simulink model "TosAeb"  (R2021a)
settings: Solver=FixedStepDiscrete  StartTime=0.0  StopTime=inf  FixedStep=0.01  RelTol=1e-3
undefined symbols (not defined in the file or in its folder): ACCModSts, AccSts1, AEB_ObjectMovingOrientation, AEB_ObjectMovingState, CN_BTCActive, CN_CurrentGearR, CN_CurrentGearR_DHT, CN_PTCActive, CN_VDCActive, CN_VSC_EspOff, CN_VSC_FCW_AEB_Error, CN_VSC_FCW_AEB_NoDispay, CN_VSC_FCW_AEB_PerfmDgdtn, CN_VSC_FCW_AEB_TempUnAvlbl, CN_VSC_VehStandstill, CNaccelAvoidMax, CNaccelAvoidMin, CNbrakePedalPress, CNcomfortStrLeftAccelMax, CNcomfortStrLeftAccelMin, CNcomfortStrRightAccelMax, CNcomfortStrRightAccelMin, CNDrvDoorOpen, CNDrvModeEco, CNDrvModeNorm, CNDrvModeOther, CNDrvModeSport, CNDrvSBRreminder, CNNull, CNobjInvalidID, CNPassDoorOpen, CNPassSBRreminder, CNPEBSAccelNegInf, CNPEBSAccelNull, CNPEBSTimeInf, CNPEBSTimeNull, CNSTOPMAX, CNSTOPNULL, CNsubjWidth, CNSwtOFF, CNSwtON, CNTTCMAX, CNTTCNULL, CNVehConfigAEBCycNone, CNVehConfigAEBNone, CNVehConfigAEBPedNone, CNVehConfigFCWNone, CNVehConfigJANone, CollisionSeverity, FALSE, FCWSnvty, JACycObjCalcInfoDefaultParams, JAPedObjCalcInfoDefaultParams, JAVehObjCalcInfoDefaultParams, latCycObjCalcInfoDefaultParams, latPedObjCalcInfoDefaultParams, longObjCalcInfoDefaultParams, ModSts, movingStatus, ObjectType ... (+1112)
libraries: simulink x3031, AEBSFunctionLib x50, Ta_Library x33, AEBSStateMachineLib x23, Mylibrary x12, simulink_extras x7, ObjSelect_lib x3, dspstat3 x2
(part 55 of 102)

== system TosAeb/FcwAebJa_Step_sys/ALG_AEBSystem/ALG_AEBController/AEB_FuncImpManagementApp/FuncManeuArbCalc/brkFuncManeuArbCalc/axTgtReqValueCalc1/axTgtReqValueCalc4AebControl/aDesiredCalc ==
  AebActiveFirstCycle : Inport
  subjVel : Inport  Port=2
  axAbsObj : Inport  Port=3
  dxFrontBumperPredicted : Inport  Port=4
  vxAbsObjPredicted : Inport  Port=5
  vxPredicted : Inport  Port=6
  dxSecureAtStart : Inport  Port=7
  accelMin : Inport  Port=8
  Bus Creator : BusCreator  Inputs=8
  Bus Selector : BusSelector  OutputSignals=dxFrontBumperPredicted,dxSecure,vxPredicted,subjVel
  Bus Selector1 : BusSelector  OutputSignals=vxPredicted,axAbsObj,vxAbsObjPredicted,dxFrontBumperPredicted,subjVel,dxSecure
  Bus Selector2 : BusSelector  OutputSignals=accelMin
  Bus Selector3 : BusSelector  OutputSignals=AebActiveFirstCycle
  If : If  IfExpression=u1
  If Action Subsystem : Subsystem  TreatAsAtomicUnit=on
  If Action Subsystem1 : Subsystem  TreatAsAtomicUnit=on
  Merge : Merge
  accelAvoidFilt : Subsystem
  underDxSecure : Subsystem
  aDesired : Outport
  wires:
  accelAvoidFilt/1(aDesired) -> aDesired/1
  Merge/1 -> accelAvoidFilt/2(accelAvoid)  "accelAvoidRaw"
  vxPredicted/1 -> Bus Creator/4  "vxPredicted"
  vxAbsObjPredicted/1 -> Bus Creator/3  "vxAbsObjPredicted"
  accelMin/1 -> Bus Creator/6  "accelMin"
  subjVel/1 -> Bus Creator/8  "subjVel"
  axAbsObj/1 -> Bus Creator/7  "axAbsObj"
  Bus Selector2/1 -> If Action Subsystem/1(accelMin)  "<accelMin>"
  Bus Selector/2 -> underDxSecure/2(dxSecure)  "<dxSecure>"
  Bus Selector/1 -> underDxSecure/1(dxFrontBumperPredicted)  "<dxFrontBumperPredicted>"
  Bus Creator/1 -> Bus Selector1/1, Bus Selector2/1, Bus Selector/1, Bus Selector3/1
  dxSecureAtStart/1 -> Bus Creator/5  "dxSecure"
  dxFrontBumperPredicted/1 -> Bus Creator/2  "dxFrontBumperPredicted"
  If/2 -> If Action Subsystem1/Action
  If Action Subsystem1/1(accelAvoid) -> Merge/2
  If Action Subsystem/1(accelAvoid) -> Merge/1
  If/1 -> If Action Subsystem/Action
  underDxSecure/1(underDxSecure) -> If/1
  Bus Selector1/1 -> If Action Subsystem1/1(vx)  "<vxPredicted>"
  Bus Selector1/3 -> If Action Subsystem1/3(vxAbsObj)  "<vxAbsObjPredicted>"
  Bus Selector1/4 -> If Action Subsystem1/4(dxFrontBumper)  "<dxFrontBumperPredicted>"
  Bus Selector1/5 -> If Action Subsystem1/5(subjVel)  "<subjVel>"
  Bus Selector1/6 -> If Action Subsystem1/6(dxSecure)  "<dxSecure>"
  AebActiveFirstCycle/1 -> Bus Creator/1
  Bus Selector3/1 -> accelAvoidFilt/1(AebActiveFirstCycle)  "<AebActiveFirstCycle>"
  Bus Selector1/2 -> If Action Subsystem1/2(axAbsObj)  "<axAbsObj>"
  Bus Selector/3 -> underDxSecure/3(vxPredicted)  "<vxPredicted>"
  Bus Selector/4 -> underDxSecure/4(subjVel)  "<subjVel>"

== system TosAeb/FcwAebJa_Step_sys/ALG_AEBSystem/ALG_AEBController/AEB_FuncImpManagementApp/FuncManeuArbCalc/brkFuncManeuArbCalc/axTgtReqValueCalc1/axTgtReqValueCalc4AebControl/axTgtReqValueCalc ==
  vx : Inport
  subjLongAccel : Inport  Port=2
  axTgtReqValueK1 : Inport  Port=3
  dxFrontBumperPredicted : Inport  Port=4
  accelMax : Inport  Port=5
  accelMin : Inport  Port=6
  JerkFuncInc : Inport  Port=7
  aDesired : Inport  Port=8
  JerkFuncDec : Inport  Port=9
  Bus Creator : BusCreator  Inputs=6
  Bus Selector : BusSelector  OutputSignals=dxFrontBumperPredicted,JerkFuncDec,aDesired,accelMax,accelMin
  Bus Selector1 : BusSelector  OutputSignals=JerkFuncInc,JerkFuncDec,aDesired,accelMax,accelMin
  If : If  IfExpression=u1
  Merge : Merge
  axTgtReqValueFinal : Subsystem
      settings: UndoDeleteFcn=Stateflow.SLINSF.SimulinkMan.restorePreDeleteFcn  PreDeleteFcn=Stateflow.SLINSF.SimulinkMan.removePreDeleteFcn
  axTgtReqValueFinal1 : Subsystem
      settings: UndoDeleteFcn=Stateflow.SLINSF.SimulinkMan.restorePreDeleteFcn  PreDeleteFcn=Stateflow.SLINSF.SimulinkMan.removePreDeleteFcn
  velReduceFlgCalc : Subsystem
  axTgtReqValue : Outport
  wires:
  Bus Selector1/5 -> axTgtReqValueFinal/6(accelMin)  "<accelMin>"
  Bus Selector1/4 -> axTgtReqValueFinal/5(accelMax)  "<accelMax>"
  Bus Selector1/3 -> axTgtReqValueFinal/4(aDesired)  "<aDesired>"
  Bus Selector1/2 -> axTgtReqValueFinal/3(JerkFuncDec)  "<JerkFuncDec>"
  Bus Selector1/1 -> axTgtReqValueFinal/2(JerkFuncInc)  "<JerkFuncInc>"
  [axTgtReqValueK1Filt] -> axTgtReqValueFinal/1(axTgtReqValueK1)
  Bus Selector/5 -> axTgtReqValueFinal1/6(accelMin)  "<accelMin>"
  Bus Selector/4 -> axTgtReqValueFinal1/5(accelMax)  "<accelMax>"
  Bus Selector/3 -> axTgtReqValueFinal1/4(aDesired)  "<aDesired>"
  Bus Selector/2 -> axTgtReqValueFinal1/3(JerkFuncDec)  "<JerkFuncDec>"
  Bus Selector/1 -> axTgtReqValueFinal1/2(JerkFuncInc)  "<dxFrontBumperPredicted>"
  Bus Creator/1 -> Bus Selector1/1, Bus Selector/1
  aDesired/1 -> Bus Creator/6  "aDesired"
  JerkFuncDec/1 -> Bus Creator/5  "JerkFuncDec"
  JerkFuncInc/1 -> Bus Creator/4  "JerkFuncInc"
  dxFrontBumperPredicted/1 -> Bus Creator/1  "dxFrontBumperPredicted"
  Merge/1 -> axTgtReqValue/1
  axTgtReqValueFinal/1(axTgtReqValue) -> Merge/2
  axTgtReqValueFinal1/1(axTgtReqValue) -> Merge/1
  If/2 -> axTgtReqValueFinal/Action
  velReduceFlgCalc/2(axTgtReqValueK1Filt) -> axTgtReqValueFinal1/1(axTgtReqValueK1), [axTgtReqValueK1Filt]
  If/1 -> axTgtReqValueFinal1/Action
  velReduceFlgCalc/1(velReduceFlg) -> If/1
  vx/1 -> velReduceFlgCalc/1(vx)
  subjLongAccel/1 -> velReduceFlgCalc/2(subjLongAccel)
  axTgtReqValueK1/1 -> velReduceFlgCalc/3(axTgtReqValueK1)
  accelMax/1 -> Bus Creator/2  "accelMax"
  accelMin/1 -> Bus Creator/3  "accelMin"

== system TosAeb/FcwAebJa_Step_sys/ALG_AEBSystem/ALG_AEBController/AEB_FuncImpManagementApp/FuncManeuArbCalc/brkFuncManeuArbCalc/axTgtReqValueCalc1/axTgtReqValueCalc4AebControl/axTgtReqValueOpt ==
  axTgtReqValueK1 : Inport
  AebFullFuncStatus : Inport  Port=2
  axTgtReqValue : Inport  Port=3
  Compare To Constant : simulink/Logic and Bit Operations/Compare To Constant  relop===  const=ModSts.StandstillActiveState  OutDataTypeStr=boolean
  Switch : Switch  Criteria=u2 ~= 0
  axTgtReqValueOpt : Outport
  wires:
  Switch/1 -> axTgtReqValueOpt/1
  AebFullFuncStatus/1 -> Compare To Constant/1
  axTgtReqValueK1/1 -> Switch/1
  axTgtReqValue/1 -> Switch/3
  Compare To Constant/1 -> Switch/2

== system TosAeb/FcwAebJa_Step_sys/ALG_AEBSystem/ALG_AEBController/AEB_FuncImpManagementApp/FuncManeuArbCalc/brkFuncManeuArbCalc/axTgtReqValueCalc1/axTgtReqValueCalc4AebFull/BrkFuncDataManagement/AEB_TgtDecelFunc ==
  maxDecel : Inport  OutDataTypeStr=single
  maxDecelGredient : Inport  Port=2  OutDataTypeStr=single
  axTgtReqValueK1 : Inport  Port=3  OutDataTypeStr=single
  AEB_TgtDecelFunc : TriggerPort  TriggerType=function-call  VariantControl=Variant
      settings: CopyFcn=Stateflow.SLINSF.SimulinkMan.removeBlockPreDeleteFcn  PreDeleteFcn=Stateflow.SLINSF.SimulinkMan.unsafePredeleteFcn
  Add : Sum  OutDataTypeStr=single
  Constant : Constant  Value=Ts
  Constant1 : Constant  Value=-12  OutDataTypeStr=single
  Constant2 : Constant  Value=CNNull  OutDataTypeStr=single
  Min : MinMax  Inputs=2
  MinMax : MinMax  Function=max  Inputs=2  OutDataTypeStr=single
  MinMax1 : MinMax  Function=max  Inputs=2  OutDataTypeStr=single
  Product : Product  OutDataTypeStr=single
  axTgtReqValue : Outport
  wires:
  maxDecelGredient/1 -> Product/2
  Constant1/1 -> MinMax1/2
  MinMax/1 -> MinMax1/1
  Product/1 -> Add/1
  Add/1 -> MinMax/1
  Constant/1 -> Product/1
  Min/1 -> axTgtReqValue/1
  maxDecel/1 -> MinMax/2
  axTgtReqValueK1/1 -> Add/2
  MinMax1/1 -> Min/1
  Constant2/1 -> Min/2

== system TosAeb/FcwAebJa_Step_sys/ALG_AEBSystem/ALG_AEBController/AEB_FuncImpManagementApp/FuncManeuArbCalc/brkFuncManeuArbCalc/axTgtReqValueCalc1/axTgtReqValueCalc4AebFull/BrkFuncDataManagement/VehAEBfullMaxDecelLookupTable ==
  subjVel : Inport  OutDataTypeStr=single
  f : TriggerPort  TriggerType=function-call  VariantControl=Variant
      settings: CopyFcn=Stateflow.SLINSF.SimulinkMan.removeBlockPreDeleteFcn  PreDeleteFcn=Stateflow.SLINSF.SimulinkMan.unsafePredeleteFcn
  MaxDecelLookupTable : Lookup_n-D  NumberOfTableDimensions=1  BreakpointsForDimension1=P_AEB_FIM_VehAEBfullDecelMinThresMOX  BeginIndexSearchUsingPreviousIndexResult=on  Table=P_AEB_FIM_VehAEBfullDecelMinThresMOY  ExtrapMethod=Clip  InternalRulePriority=Speed  OutDataTypeStr=single  RndMeth=Simplest  InputSameDT=on
  MaxDecel : Outport
  wires:
  MaxDecelLookupTable/1 -> MaxDecel/1
  subjVel/1 -> MaxDecelLookupTable/1

== system TosAeb/FcwAebJa_Step_sys/ALG_AEBSystem/ALG_AEBController/AEB_FuncImpManagementApp/FuncManeuArbCalc/brkFuncManeuArbCalc/axTgtReqValueCalc1/axTgtReqValueCalc4AebFull/BrkFuncDataManagement/VehAEBpartMaxDecelLookupTable ==
  subjVel : Inport  OutDataTypeStr=single
  f : TriggerPort  TriggerType=function-call  VariantControl=Variant
      settings: CopyFcn=Stateflow.SLINSF.SimulinkMan.removeBlockPreDeleteFcn  PreDeleteFcn=Stateflow.SLINSF.SimulinkMan.unsafePredeleteFcn
  MaxDecelLookupTable : Lookup_n-D  NumberOfTableDimensions=1  BreakpointsForDimension1=P_AEB_FIM_VehAEBpartDecelMinThresX  BeginIndexSearchUsingPreviousIndexResult=on  Table=P_AEB_FIM_VehAEBpartDecelMinThresY  ExtrapMethod=Clip  InternalRulePriority=Speed  OutDataTypeStr=single  RndMeth=Simplest  InputSameDT=on
  MaxDecel : Outport
  wires:
  MaxDecelLookupTable/1 -> MaxDecel/1
  subjVel/1 -> MaxDecelLookupTable/1

== system TosAeb/FcwAebJa_Step_sys/ALG_AEBSystem/ALG_AEBController/AEB_JaObjCalcApp/JAPedTgtInfoCalc/JAPedCollisonAssessInfo/LatPedCollisonAssessInfoCalc/PedDistBrakeFullCalc/BrkSys ==
  subjVel : Inport
  subjLongAccel : Inport  Port=2  OutDataTypeStr=single
  BrkJerkMaxFullBrakeLookupTable : Lookup_n-D  NumberOfTableDimensions=1  BreakpointsForDimension1=P_AEB_JaPedCA_BrkJerkMaxFullBrakeX  Table=P_AEB_JaPedCA_BrkJerkMaxFullBrakeY  ExtrapMethod=Clip  InternalRulePriority=Speed  RndMeth=Simplest  InputSameDT=on
  DecelMaxLookupTable : Lookup_n-D  NumberOfTableDimensions=1  BreakpointsForDimension1=P_AEB_JaPedCA_DecelMaxFullBrakeX  Table=P_AEB_JaPedCA_DecelMaxFullBrakeY  ExtrapMethod=Clip  InternalRulePriority=Speed  RndMeth=Simplest  InputSameDT=on
  MinMax : MinMax  Function=max  Inputs=2  OutDataTypeStr=single
  PKjerk : Constant  Commented=on  Value=P_AEB_JaPedCA_BrkJerkMaxFullBrake  OutDataTypeStr=single
  PaccelMax1 : Constant  Commented=on  Value=P_AEB_JaPedCA_DecelMaxFullBrake  OutDataTypeStr=single
  PtDelay : Constant  Value=P_AEB_JaPedCA_BrkSysDelayTimeFullBrake  OutDataTypeStr=single
  PvelDelta : Constant  Value=P_AEB_JaPedCA_VelReduceMaxFullBrake  OutDataTypeStr=single
  tDelay : Outport
  Kjerk : Outport  Port=2
  velDelta : Outport  Port=3
  accelMax : Outport  Port=4
  subjLongAccelFilt : Outport  Port=5
  wires:
  PtDelay/1 -> tDelay/1
  BrkJerkMaxFullBrakeLookupTable/1 -> Kjerk/1
  PvelDelta/1 -> velDelta/1
  DecelMaxLookupTable/1 -> accelMax/1, MinMax/1
  subjLongAccel/1 -> MinMax/2
  MinMax/1 -> subjLongAccelFilt/1
  subjVel/1 -> BrkJerkMaxFullBrakeLookupTable/1, DecelMaxLookupTable/1

== system TosAeb/FcwAebJa_Step_sys/ALG_AEBSystem/ALG_AEBController/AEB_JaObjCalcApp/JAPedTgtInfoCalc/JAPedCollisonAssessInfo/LatPedCollisonAssessInfoCalc/PedDistBrakeFullCalc/DistPhase1Calc ==
  subjVel : Inport  OutDataTypeStr=single
  tPhase1 : Inport  Port=2  OutDataTypeStr=single
  subjLongAccelFilt : Inport  Port=3  OutDataTypeStr=single
  Add : Sum  OutDataTypeStr=single
  Constant : Constant  Value=0.5  OutDataTypeStr=single
  Product : Product  Inputs=4  OutDataTypeStr=single
  Product1 : Product  OutDataTypeStr=single
  distPhase1 : Outport
  wires:
  tPhase1/1 -> Product1/2, Product/2, Product/1
  subjLongAccelFilt/1 -> Product/3
  Constant/1 -> Product/4
  subjVel/1 -> Product1/1
  Product1/1 -> Add/1
  Product/1 -> Add/2
  Add/1 -> distPhase1/1

== system TosAeb/FcwAebJa_Step_sys/ALG_AEBSystem/ALG_AEBController/AEB_JaObjCalcApp/JAPedTgtInfoCalc/JAPedCollisonAssessInfo/LatPedCollisonAssessInfoCalc/PedDistBrakeFullCalc/DistPhase2Calc ==
  subjVel : Inport  OutDataTypeStr=single
  Kjerk : Inport  Port=2  OutDataTypeStr=single
  tPhase1 : Inport  Port=3  OutDataTypeStr=single
  subjLongAccelFilt : Inport  Port=4  OutDataTypeStr=single
  accelMax : Inport  Port=5  OutDataTypeStr=single
  distPhase2Calc : Subsystem
  tPhase2Calc : Subsystem
  distPhase2 : Outport
  egoStopFlg : Outport  Port=2
  tJerkWork : Outport  Port=3
  tPhase2 : Outport  Port=4
  wires:
  subjLongAccelFilt/1 -> tPhase2Calc/3(subjLongAccelFilt), distPhase2Calc/3(subjLongAccelFilt)
  Kjerk/1 -> tPhase2Calc/2(Kjerk), distPhase2Calc/4(Kjerk)
  subjVel/1 -> distPhase2Calc/5(subjVel), tPhase2Calc/1(subjVel)
  accelMax/1 -> tPhase2Calc/4(accelMax)
  tPhase2Calc/3(tPhase2) -> tPhase2/1, distPhase2Calc/1(tPhase2)
  distPhase2Calc/1(distPhase2) -> distPhase2/1
  tPhase2Calc/2(tJerkWork) -> tJerkWork/1
  tPhase2Calc/1(egoStopFlg) -> egoStopFlg/1
  tPhase1/1 -> distPhase2Calc/2(tPhase1)

== system TosAeb/FcwAebJa_Step_sys/ALG_AEBSystem/ALG_AEBController/AEB_JaObjCalcApp/JAPedTgtInfoCalc/JAPedCollisonAssessInfo/LatPedCollisonAssessInfoCalc/PedDistBrakeFullCalc/DistPhase3Calc ==
  egoStopFlg : Inport
  tJerkWork : Inport  Port=2  OutDataTypeStr=single
  tPhase2 : Inport  Port=3  OutDataTypeStr=single
  tPhase1 : Inport  Port=4  OutDataTypeStr=single
  velDelta : Inport  Port=5  OutDataTypeStr=single
  subjVel : Inport  Port=6  OutDataTypeStr=single
  subjLongAccelFilt : Inport  Port=7  OutDataTypeStr=single
  Kjerk : Inport  Port=8  OutDataTypeStr=single
  accelMax : Inport  Port=9  OutDataTypeStr=single
  Bus Creator : BusCreator  Inputs=8
  Bus Selector : BusSelector  OutputSignals=subjVel,subjLongAccelFilt,tJerkWork,Kjerk,accelMax,velDelta
  Bus Selector1 : BusSelector  OutputSignals=subjVel,subjLongAccelFilt,Kjerk,tPhase1,tPhase2,accelMax
  If : If  IfExpression=u1
  Merge : Merge
  distPhase3Calc : Subsystem
  tPhase3Calc : Subsystem  TreatAsAtomicUnit=on
  tPhase3Calc1 : Subsystem  TreatAsAtomicUnit=on
  distPhase3 : Outport
  tPhase3 : Outport  Port=2
  wires:
  If/1 -> tPhase3Calc1/Action
  egoStopFlg/1 -> If/1
  If/2 -> tPhase3Calc/Action
  tPhase3Calc1/1(tPhase3) -> Merge/1
  tPhase3Calc/1(tPhase3) -> Merge/2
  Merge/1 -> tPhase3/1, distPhase3Calc/1(tPhase3)
  distPhase3Calc/1(distPhase3) -> distPhase3/1
  accelMax/1 -> Bus Creator/8  "accelMax"
  Kjerk/1 -> Bus Creator/7  "Kjerk"
  subjLongAccelFilt/1 -> Bus Creator/6  "subjLongAccelFilt"
  subjVel/1 -> Bus Creator/5  "subjVel"
  tJerkWork/1 -> Bus Creator/1  "tJerkWork"
  tPhase1/1 -> Bus Creator/2  "tPhase1"
  tPhase2/1 -> Bus Creator/3  "tPhase2"
  velDelta/1 -> Bus Creator/4  "velDelta"
  Bus Creator/1 -> Bus Selector1/1, Bus Selector/1
  Bus Selector/3 -> tPhase3Calc/3(tJerkWork)  "<tJerkWork>"
  Bus Selector/4 -> tPhase3Calc/4(Kjerk)  "<Kjerk>"
  Bus Selector/2 -> tPhase3Calc/2(subjLongAccelFilt)  "<subjLongAccelFilt>"
  Bus Selector/5 -> tPhase3Calc/5(accelMax)  "<accelMax>"
  Bus Selector/1 -> tPhase3Calc/1(subjVel)  "<subjVel>"
  Bus Selector/6 -> tPhase3Calc/6(velDelta)  "<velDelta>"
  Bus Selector1/6 -> distPhase3Calc/7(accelMax)  "<accelMax>"
  Bus Selector1/5 -> distPhase3Calc/6(tPhase2)  "<tPhase2>"
  Bus Selector1/4 -> distPhase3Calc/5(tPhase1)  "<tPhase1>"
  Bus Selector1/3 -> distPhase3Calc/4(Kjerk)  "<Kjerk>"
  Bus Selector1/2 -> distPhase3Calc/3(subjLongAccelFilt)  "<subjLongAccelFilt>"
  Bus Selector1/1 -> distPhase3Calc/2(subjVel)  "<subjVel>"

== system TosAeb/FcwAebJa_Step_sys/ALG_AEBSystem/ALG_AEBController/AEB_JaObjCalcApp/JAPedTgtInfoCalc/JAPedCollisonAssessInfo/LatPedCollisonAssessInfoCalc/PedDistBrakeFullCalc/distBrakeCalc ==
  distPhase1 : Inport  OutDataTypeStr=single
  distPhase2 : Inport  Port=2  OutDataTypeStr=single
  distPhase3 : Inport  Port=3  OutDataTypeStr=single
  Add : Sum  Inputs=3  OutDataTypeStr=single
  distBrake : Outport
  wires:
  distPhase2/1 -> Add/2
  distPhase1/1 -> Add/1
  distPhase3/1 -> Add/3
  Add/1 -> distBrake/1

== system TosAeb/FcwAebJa_Step_sys/ALG_AEBSystem/ALG_AEBController/AEB_JaObjCalcApp/JAPedTgtInfoCalc/JAPedCollisonAssessInfo/LatPedCollisonAssessInfoCalc/PedDistBrakeFullCalc/tBrakeCalc ==
  tPhase3 : Inport  OutDataTypeStr=single
  tPhase1 : Inport  Port=2  OutDataTypeStr=single
  tPhase2 : Inport  Port=3  OutDataTypeStr=single
  Add : Sum  Inputs=+++  OutDataTypeStr=single
  tBrake : Outport
  wires:
  tPhase2/1 -> Add/2
  tPhase3/1 -> Add/3
  tPhase1/1 -> Add/1
  Add/1 -> tBrake/1

== system TosAeb/FcwAebJa_Step_sys/ALG_AEBSystem/ALG_AEBController/AEB_JaObjCalcApp/JAPedTgtInfoCalc/JAPedCollisonAssessInfo/LatPedCollisonAssessInfoCalc/VelAbsTgtCorrCalc/RadicalRelevantCalc ==
  dy : Inport  OutDataTypeStr=single
  dxFrontBumper : Inport  Port=2  OutDataTypeStr=single
  subjYawRate : Inport  Port=3  OutDataTypeStr=single
  Bus Creator : BusCreator  Inputs=2
  Math Function : Math  Operator=hypot  SignedPower=on  SaturateOnIntegerOverflow=on
  Product1 : Product  OutDataTypeStr=single
  Sincos : Trigonometry  Operator=sincos
  Trigonometric Function : Trigonometry  Operator=atan2
  radialVelRel : Outport
  sincosCoef : Outport  Port=2
  wires:
  subjYawRate/1 -> Product1/1
  Math Function/1 -> Product1/2  "dvRadial"
  Product1/1 -> radialVelRel/1  "radialVelRel"
  dy/1 -> Math Function/2, Trigonometric Function/1
  dxFrontBumper/1 -> Trigonometric Function/2, Math Function/1
  Trigonometric Function/1 -> Sincos/1
  Sincos/2 -> Bus Creator/2  "cosCoef"
  Bus Creator/1 -> sincosCoef/1  "sincosCoef"
  Sincos/1 -> Bus Creator/1  "sinCoef"

== system TosAeb/FcwAebJa_Step_sys/ALG_AEBSystem/ALG_AEBController/AEB_JaObjCalcApp/JAPedTgtInfoCalc/JAPedCollisonAssessInfo/LatPedCollisonAssessInfoCalc/VelAbsTgtCorrCalc/RadicalToXYTrans ==
  velRadicalXY : Inport
  sincosCoef : Inport  Port=2
  subjVel : Inport  Port=3  OutDataTypeStr=single
  Add3 : Sum  Inputs=++-  OutDataTypeStr=single
  Add4 : Sum  OutDataTypeStr=single
  Bus Creator4 : BusCreator  Inputs=2
  Bus Selector10 : BusSelector  OutputSignals=velRadicalXY.velRadicalY
  Bus Selector11 : BusSelector  OutputSignals=sincosCoef.sinCoef
  Bus Selector12 : BusSelector  OutputSignals=velRadicalXY.velRadicalX
  Bus Selector13 : BusSelector  OutputSignals=sincosCoef.sinCoef
  Bus Selector14 : BusSelector  OutputSignals=velRadicalXY.velRadicalY
  Bus Selector15 : BusSelector  OutputSignals=sincosCoef.cosCoef
  Bus Selector8 : BusSelector  OutputSignals=velRadicalXY.velRadicalX
  Bus Selector9 : BusSelector  OutputSignals=sincosCoef.cosCoef
  Product{7..10} (4 alike) : Product  OutDataTypeStr=single
  vxAbsTargetCorr : Outport
  vyAbsTargetCorr : Outport  Port=2
  wires:
  Product8/1 -> Add3/3
  Bus Selector13/1 -> Product9/2  "<sinCoef>"
  Add3/1 -> vxAbsTargetCorr/1  "vxAbsTargetCorr"
  Product7/1 -> Add3/2
  Bus Selector14/1 -> Product10/1  "<velRadicalY>"
  Bus Selector11/1 -> Product8/2  "<sinCoef>"
  Bus Selector10/1 -> Product8/1  "<velRadicalY>"
  subjVel/1 -> Add3/1
  Add4/1 -> vyAbsTargetCorr/1  "vyAbsTargetCorr"
  Bus Creator4/1 -> Bus Selector15/1, Bus Selector14/1, Bus Selector13/1, Bus Selector12/1, Bus Selector11/1, Bus Selector10/1, Bus Selector9/1, Bus Selector8/1
  Bus Selector9/1 -> Product7/2  "<cosCoef>"
  Product10/1 -> Add4/2
  Bus Selector15/1 -> Product10/2  "<cosCoef>"
  Bus Selector8/1 -> Product7/1  "<velRadicalX>"
  Product9/1 -> Add4/1
  Bus Selector12/1 -> Product9/1  "<velRadicalX>"
  velRadicalXY/1 -> Bus Creator4/1
  sincosCoef/1 -> Bus Creator4/2

== system TosAeb/FcwAebJa_Step_sys/ALG_AEBSystem/ALG_AEBController/AEB_JaObjCalcApp/JAPedTgtInfoCalc/JAPedCollisonAssessInfo/LatPedCollisonAssessInfoCalc/VelAbsTgtCorrCalc/XYToRadicalTrans ==
  LongLatVelRel : Inport
  radialVelRel : Inport  Port=2  OutDataTypeStr=single
  sincosCoef : Inport  Port=3
  Add : Sum  OutDataTypeStr=single
  Add1 : Sum  OutDataTypeStr=single
  Add2 : Sum  Inputs=+-  OutDataTypeStr=single
  Bus Creator2 : BusCreator  Inputs=2
  Bus Creator3 : BusCreator  Inputs=2
  Bus Selector : BusSelector  OutputSignals=LongLatVelRel.vx
  Bus Selector1 : BusSelector  OutputSignals=sincosCoef.cosCoef
  Bus Selector2 : BusSelector  OutputSignals=LongLatVelRel.vy
  Bus Selector3 : BusSelector  OutputSignals=sincosCoef.sinCoef
  Bus Selector4 : BusSelector  OutputSignals=LongLatVelRel.vy
  Bus Selector5 : BusSelector  OutputSignals=sincosCoef.cosCoef
  Bus Selector6 : BusSelector  OutputSignals=LongLatVelRel.vx
  Bus Selector7 : BusSelector  OutputSignals=sincosCoef.sinCoef
  Product{2,4..6} (4 alike) : Product  OutDataTypeStr=single
  velRadicalXY : Outport
  wires:
  Product6/1 -> Add2/2
  Bus Creator3/1 -> velRadicalXY/1  "velRadicalXY"
  Product5/1 -> Add2/1
  Bus Selector2/1 -> Product4/1  "<vy>"
  Product4/1 -> Add/2
  Product2/1 -> Add/1
  Bus Creator2/1 -> Bus Selector7/1, Bus Selector6/1, Bus Selector5/1, Bus Selector4/1, Bus Selector3/1, Bus Selector2/1, Bus Selector1/1, Bus Selector/1
  Bus Selector5/1 -> Product5/2  "<cosCoef>"
  Add1/1 -> Bus Creator3/2  "velRadicalY"
  Bus Selector6/1 -> Product6/1  "<vx>"
  Add/1 -> Bus Creator3/1  "velRadicalX"
  Bus Selector4/1 -> Product5/1  "<vy>"
  Bus Selector1/1 -> Product2/2  "<cosCoef>"
  radialVelRel/1 -> Add1/2
  Bus Selector3/1 -> Product4/2  "<sinCoef>"
  Bus Selector/1 -> Product2/1  "<vx>"
  Bus Selector7/1 -> Product6/2  "<sinCoef>"
  sincosCoef/1 -> Bus Creator2/2
  LongLatVelRel/1 -> Bus Creator2/1
  Add2/1 -> Add1/1

== system TosAeb/FcwAebJa_Step_sys/ALG_AEBSystem/ALG_AEBController/AEB_JaObjCalcApp/JAPedTgtInfoCalc/JAPedCollisonAssessInfo/LatPedCollisonAssessInfoCalc/tBrakeingCalc/tBrakeCalc ==
  tBarke : Inport
  TTB : Outport
  wires:
  tBarke/1 -> TTB/1

== system TosAeb/FcwAebJa_Step_sys/ALG_AEBSystem/ALG_AEBController/AEB_JaObjCalcApp/JAPedTgtInfoCalc/JAPedCollisonAssessInfo/LatPedCollisonAssessInfoCalc/tBrakeingCalc/tBrakeLookup ==
  subjVel : Inport  OutDataTypeStr=single
  1-D Lookup Table : Lookup_n-D  NumberOfTableDimensions=1  BreakpointsForDimension1=P_JaPedCA_SubjVelBrkWorkTimeX  Table=P_JaPedCA_BrkWorkTimeX  ExtrapMethod=Clip  InternalRulePriority=Speed  OutDataTypeStr=single  RndMeth=Simplest  InputSameDT=on
  TTB : Outport
  wires:
  1-D Lookup Table/1 -> TTB/1
  subjVel/1 -> 1-D Lookup Table/1

== system TosAeb/FcwAebJa_Step_sys/ALG_AEBSystem/ALG_AEBController/AEB_JaObjCalcApp/JAPedTgtInfoCalc/JAPedObjCalcInfoCalc/JAPedObjInfoCalc/ObjCrossFlgCal/CollisionProbCalc ==
  tPredTime : Inport  OutDataTypeStr=single
  subjVel : Inport  Port=2  OutDataTypeStr=single
  subjLongAccel : Inport  Port=3  OutDataTypeStr=single
  subjLatAccel : Inport  Port=4  OutDataTypeStr=single
  subjYawRate : Inport  Port=5  OutDataTypeStr=single
  ObjectMovingOrientation : Inport  Port=6
  ObjectLength : Inport  Port=7  OutDataTypeStr=single
  ObjectTypeCameraConfirmed : Inport  Port=8
  ObjectWidth : Inport  Port=9  OutDataTypeStr=single
  dx : Inport  Port=10  OutDataTypeStr=single
  dy : Inport  Port=11  OutDataTypeStr=single
  ObjectID : Inport  Port=12
  vyRelCorr : Inport  Port=13  OutDataTypeStr=single
  vxAbsObj : Inport  Port=14  OutDataTypeStr=single
  ObjectYawAngleCorr : Inport  Port=15  OutDataTypeStr=single
  function : TriggerPort  TriggerType=function-call  VariantControl=Variant  PortDimensions=1
  If : If  IfExpression=u1
  If Action Subsystem : Subsystem  TreatAsAtomicUnit=on
  Merge : Merge
  ObjIsInvalidCalc : Subsystem
  Subsystem : Subsystem
  ObjColliFlg : Outport
  wires:
  tPredTime/1 -> Subsystem/1(tPredTime)
  subjVel/1 -> Subsystem/2(subjVel)
  subjLongAccel/1 -> Subsystem/3(subjLongAccel)
  subjLatAccel/1 -> Subsystem/4(subjLatAccel)
  subjYawRate/1 -> Subsystem/5(subjYawRate)
  ObjectYawAngleCorr/1 -> Subsystem/6(ObjectYawAngleCorr)
  ObjectLength/1 -> Subsystem/8(ObjectLength)
  ObjectTypeCameraConfirmed/1 -> Subsystem/9(ObjectTypeCameraConfirmed)
  ObjectWidth/1 -> Subsystem/10(ObjectWidth)
  dx/1 -> Subsystem/11(dx)
  vxAbsObj/1 -> Subsystem/14(vxAbsObj)
  If/1 -> If Action Subsystem/Action
  If Action Subsystem/1(ObjColliFlg) -> Merge/1
  If/2 -> Subsystem/Action
  Merge/1 -> ObjColliFlg/1
  Subsystem/1(ObjColliFlg) -> Merge/2
  dy/1 -> Subsystem/12(dy)
  ObjectID/1 -> ObjIsInvalidCalc/1(ObjectID)
  vyRelCorr/1 -> Subsystem/13(vyRelCorr)
  ObjIsInvalidCalc/1(ObjIsInvalid) -> If/1
  ObjectMovingOrientation/1 -> Subsystem/7(ObjectMovingOrientation)

== system TosAeb/FcwAebJa_Step_sys/ALG_AEBSystem/ALG_AEBController/AEB_JaObjCalcApp/JAPedTgtInfoCalc/JAPedObjCalcInfoCalc/JAPedObjInfoCalc/SensorTgtInfo2AbsTgtInfoTrans/ObjFusQualityCal/SensorDxThres ==
  subjVel : Inport
  f : TriggerPort  TriggerType=function-call  VariantControl=(inherit)
      settings: CopyFcn=Stateflow.SLINSF.SimulinkMan.removeBlockPreDeleteFcn  PreDeleteFcn=Stateflow.SLINSF.SimulinkMan.unsafePredeleteFcn
  deltaDxThresCalc : Lookup_n-D  NumberOfTableDimensions=1  BreakpointsForDimension1=P_AEB_JaPedAbs_SensorDeltDxThresX  Table=P_AEB_JaPedAbs_SensorDeltDxThresY  ExtrapMethod=Clip  InternalRulePriority=Speed  RndMeth=Simplest  InputSameDT=on
  deltaDxThres : Outport  OutDataTypeStr=single
  wires:
  subjVel/1 -> deltaDxThresCalc/1
  deltaDxThresCalc/1 -> deltaDxThres/1

== system TosAeb/FcwAebJa_Step_sys/ALG_AEBSystem/ALG_AEBController/AEB_JaObjCalcApp/JAVehTgtInfoCalc/AEBJAVehObjCalc/JAVehObjInfoCalc/ObjCrossFlgCal/CollisionProbCalc ==
  tPredTime : Inport  OutDataTypeStr=single
  subjVel : Inport  Port=2
  subjLongAccel : Inport  Port=3
  subjLatAccel : Inport  Port=4
  subjYawRate : Inport  Port=5
  ObjectYawAngle : Inport  Port=6  OutDataTypeStr=single
  ObjectMovingOrientation : Inport  Port=7
  ObjectLength : Inport  Port=8  OutDataTypeStr=single
  FusionType : Inport  Port=9
  ObjectWidth : Inport  Port=10  OutDataTypeStr=single
  dx : Inport  Port=11  OutDataTypeStr=single
  vy : Inport  Port=12  OutDataTypeStr=single
  ay : Inport  Port=13  OutDataTypeStr=single
  dy : Inport  Port=14  OutDataTypeStr=single
  ObjectID : Inport  Port=15
  vxAbsObj : Inport  Port=16  OutDataTypeStr=single
  axAbsObj : Inport  Port=17  OutDataTypeStr=single
  function : TriggerPort  TriggerType=function-call  VariantControl=Variant  PortDimensions=1
  If : If  IfExpression=u1 == 0
  If Action Subsystem : Subsystem  TreatAsAtomicUnit=on
  Merge : Merge
  Subsystem : Subsystem
  ObjColliFlg : Outport
  wires:
  tPredTime/1 -> Subsystem/1(tPredTime)
  subjVel/1 -> Subsystem/2(subjVel)
  subjLongAccel/1 -> Subsystem/3(subjLongAccel)
  subjLatAccel/1 -> Subsystem/4(subjLatAccel)
  subjYawRate/1 -> Subsystem/5(subjYawRate)
  ObjectLength/1 -> Subsystem/8(ObjectLength)
  FusionType/1 -> Subsystem/9(FusionType)
  ObjectWidth/1 -> Subsystem/10(ObjectWidth)
  dx/1 -> Subsystem/11(dx)
  vy/1 -> Subsystem/12(vy)
  ay/1 -> Subsystem/13(ay)
  dy/1 -> Subsystem/14(dy)
  vxAbsObj/1 -> Subsystem/15(vxAbsObj)
  axAbsObj/1 -> Subsystem/16(axAbsObj)
  ObjectID/1 -> If/1
  If/1 -> If Action Subsystem/Action
  If Action Subsystem/1(ObjColliFlg) -> Merge/1
  If/2 -> Subsystem/Action
  Merge/1 -> ObjColliFlg/1
  Subsystem/1(ObjColliFlg) -> Merge/2
  ObjectMovingOrientation/1 -> Subsystem/7(ObjectMovingOrientation)
  ObjectYawAngle/1 -> Subsystem/6(ObjectYawAngle)

== system TosAeb/FcwAebJa_Step_sys/ALG_AEBSystem/ALG_AEBController/AEB_JaObjCalcApp/JAVehTgtInfoCalc/AEBJAVehObjCalc/JAVehObjInfoCalc/SensorTgtInfo2AbsTgtInfoTrans/ObjFusQualityCal/SensorDxThres ==
  subjVel : Inport
  f : TriggerPort  TriggerType=function-call  VariantControl=(inherit)
      settings: CopyFcn=Stateflow.SLINSF.SimulinkMan.removeBlockPreDeleteFcn  PreDeleteFcn=Stateflow.SLINSF.SimulinkMan.unsafePredeleteFcn
  deltaDxThresCalc : Lookup_n-D  NumberOfTableDimensions=1  BreakpointsForDimension1=P_AEB_JaVehAbs_SensorDeltDxThresX  Table=P_AEB_JaVehAbs_SensorDeltDxThresY  ExtrapMethod=Clip  InternalRulePriority=Speed  RndMeth=Simplest  InputSameDT=on
  deltaDyThresCalc : Lookup_n-D  NumberOfTableDimensions=1  BreakpointsForDimension1=P_AEB_JaVehAbs_SensorDeltDyThresX  Table=P_AEB_JaVehAbs_SensorDeltDyThresY  ExtrapMethod=Clip  InternalRulePriority=Speed  RndMeth=Simplest  InputSameDT=on
  deltaDxThres : Outport  OutDataTypeStr=single
  deltaDyThres : Outport  Port=2  OutDataTypeStr=single
  wires:
  subjVel/1 -> deltaDxThresCalc/1, deltaDyThresCalc/1
  deltaDxThresCalc/1 -> deltaDxThres/1
  deltaDyThresCalc/1 -> deltaDyThres/1

== system TosAeb/FcwAebJa_Step_sys/ALG_AEBSystem/ALG_AEBController/AEB_JaObjCalcApp/JAVehTgtInfoCalc/JAVehCollisonAssessInfoCalc/JaVehCollisonAssessInfoCalc/VehDistBrakeFullCalc/BrkSys ==
  subjVel : Inport
  subjLongAccel : Inport  Port=2  OutDataTypeStr=single
  BrkJerkMaxFullBrakeLookupTable : Lookup_n-D  NumberOfTableDimensions=1  BreakpointsForDimension1=P_AEB_JaVehCA_BrkJerkMaxFullBrakeX  Table=P_AEB_JaVehCA_BrkJerkMaxFullBrakeY  ExtrapMethod=Clip  InternalRulePriority=Speed  RndMeth=Simplest  InputSameDT=on
  DecelMaxFullBrakeFullBrakeLookupTable : Lookup_n-D  NumberOfTableDimensions=1  BreakpointsForDimension1=P_AEB_JaVehCA_DecelMaxFullBrakeX  Table=P_AEB_JaVehCA_DecelMaxFullBrakeY  ExtrapMethod=Clip  InternalRulePriority=Speed  RndMeth=Simplest  InputSameDT=on
  MinMax : MinMax  Function=max  Inputs=2  OutDataTypeStr=single
  PKjerk : Constant  Commented=on  Value=P_AEB_JaVehCA_BrkJerkMaxFullBrake  OutDataTypeStr=single
  PaccelMax : Constant  Commented=on  Value=P_AEB_JaVehCA_DecelMaxFullBrake  OutDataTypeStr=single
  PtDelay : Constant  Value=P_AEB_JaVehCA_BrkSysDelayTimeFullBrake  OutDataTypeStr=single
  PvelDelta : Constant  Value=P_AEB_JaVehCA_VelReduceMaxFullBrake  OutDataTypeStr=single
  tDelay : Outport
  Kjerk : Outport  Port=2
  velDelta : Outport  Port=3
  accelMax : Outport  Port=4
  subjLongAccelFilt : Outport  Port=5
  wires:
  PtDelay/1 -> tDelay/1
  BrkJerkMaxFullBrakeLookupTable/1 -> Kjerk/1
  PvelDelta/1 -> velDelta/1
  DecelMaxFullBrakeFullBrakeLookupTable/1 -> MinMax/1, accelMax/1
  subjLongAccel/1 -> MinMax/2
  MinMax/1 -> subjLongAccelFilt/1
  subjVel/1 -> DecelMaxFullBrakeFullBrakeLookupTable/1, BrkJerkMaxFullBrakeLookupTable/1

== system TosAeb/FcwAebJa_Step_sys/ALG_AEBSystem/ALG_AEBController/AEB_JaObjCalcApp/JAVehTgtInfoCalc/JAVehCollisonAssessInfoCalc/JaVehCollisonAssessInfoCalc/VehDistBrakeFullCalc/DistPhase1Calc ==
  subjVel : Inport  OutDataTypeStr=single
  tPhase1 : Inport  Port=2  OutDataTypeStr=single
  subjLongAccelFilt : Inport  Port=3  OutDataTypeStr=single
  Add : Sum  OutDataTypeStr=single
  Constant : Constant  Value=0.5  OutDataTypeStr=single
  Product : Product  Inputs=4  OutDataTypeStr=single
  Product1 : Product  OutDataTypeStr=single
  distPhase1 : Outport
  wires:
  tPhase1/1 -> Product1/2, Product/2, Product/1
  subjLongAccelFilt/1 -> Product/3
  Constant/1 -> Product/4
  subjVel/1 -> Product1/1
  Product1/1 -> Add/1
  Product/1 -> Add/2
  Add/1 -> distPhase1/1

== system TosAeb/FcwAebJa_Step_sys/ALG_AEBSystem/ALG_AEBController/AEB_JaObjCalcApp/JAVehTgtInfoCalc/JAVehCollisonAssessInfoCalc/JaVehCollisonAssessInfoCalc/VehDistBrakeFullCalc/DistPhase2Calc ==
  subjVel : Inport  OutDataTypeStr=single
  Kjerk : Inport  Port=2  OutDataTypeStr=single
  subjLongAccelFilt : Inport  Port=3  OutDataTypeStr=single
  accelMax : Inport  Port=4  OutDataTypeStr=single
  distPhase2Calc : Subsystem
  tPhase2Calc : Subsystem
  distPhase2 : Outport
  egoStopFlg : Outport  Port=2
  tJerkWork : Outport  Port=3
  tPhase2 : Outport  Port=4
  wires:
  subjLongAccelFilt/1 -> tPhase2Calc/3(subjLongAccelFilt), distPhase2Calc/2(subjAccelFilt)
  Kjerk/1 -> tPhase2Calc/2(Kjerk), distPhase2Calc/3(Kjerk)
  subjVel/1 -> distPhase2Calc/4(subjVel), tPhase2Calc/1(subjVel)
  accelMax/1 -> tPhase2Calc/4(accelMax)
  tPhase2Calc/3(tPhase2) -> tPhase2/1, distPhase2Calc/1(tPhase2)
  distPhase2Calc/1(distPhase2) -> distPhase2/1
  tPhase2Calc/2(tJerkWork) -> tJerkWork/1
  tPhase2Calc/1(egoStopFlg) -> egoStopFlg/1

== system TosAeb/FcwAebJa_Step_sys/ALG_AEBSystem/ALG_AEBController/AEB_JaObjCalcApp/JAVehTgtInfoCalc/JAVehCollisonAssessInfoCalc/JaVehCollisonAssessInfoCalc/VehDistBrakeFullCalc/DistPhase3Calc ==
  egoStopFlg : Inport
  tJerkWork : Inport  Port=2  OutDataTypeStr=single
  tPhase2 : Inport  Port=3  OutDataTypeStr=single
  tPhase1 : Inport  Port=4  OutDataTypeStr=single
  velDelta : Inport  Port=5  OutDataTypeStr=single
  subjVel : Inport  Port=6  OutDataTypeStr=single
  subjLongAccelFilt : Inport  Port=7  OutDataTypeStr=single
  Kjerk : Inport  Port=8  OutDataTypeStr=single
  accelMax : Inport  Port=9  OutDataTypeStr=single
  Bus Creator : BusCreator  Inputs=8
  Bus Selector : BusSelector  OutputSignals=subjVel,subjLongAccelFilt,tJerkWork,Kjerk,accelMax,velDelta
  Bus Selector1 : BusSelector  OutputSignals=subjVel,subjLongAccelFilt,Kjerk,tPhase1,tPhase2,accelMax
  If : If  IfExpression=u1
  Merge : Merge
  distPhase3Calc : Subsystem
  tPhase3Calc : Subsystem  TreatAsAtomicUnit=on
  tPhase3Calc1 : Subsystem  TreatAsAtomicUnit=on
  distPhase3 : Outport
  tPhase3 : Outport  Port=2
  wires:
  If/1 -> tPhase3Calc1/Action
  egoStopFlg/1 -> If/1
  If/2 -> tPhase3Calc/Action
  tPhase3Calc1/1(tPhase3) -> Merge/1
  tPhase3Calc/1(tPhase3) -> Merge/2
  Merge/1 -> tPhase3/1, distPhase3Calc/1(tPhase3)
  distPhase3Calc/1(distPhase3) -> distPhase3/1
  accelMax/1 -> Bus Creator/8  "accelMax"
  Kjerk/1 -> Bus Creator/7  "Kjerk"
  subjLongAccelFilt/1 -> Bus Creator/6  "subjLongAccelFilt"
  subjVel/1 -> Bus Creator/5  "subjVel"
  tJerkWork/1 -> Bus Creator/1  "tJerkWork"
  tPhase1/1 -> Bus Creator/2  "tPhase1"
  tPhase2/1 -> Bus Creator/3  "tPhase2"
  velDelta/1 -> Bus Creator/4  "velDelta"
  Bus Creator/1 -> Bus Selector1/1, Bus Selector/1
  Bus Selector/3 -> tPhase3Calc/3(tJerkWork)  "<tJerkWork>"
  Bus Selector/4 -> tPhase3Calc/4(Kjerk)  "<Kjerk>"
  Bus Selector/2 -> tPhase3Calc/2(subjLongAccelFilt)  "<subjLongAccelFilt>"
  Bus Selector/5 -> tPhase3Calc/5(accelMax)  "<accelMax>"
  Bus Selector/1 -> tPhase3Calc/1(subjVel)  "<subjVel>"
  Bus Selector/6 -> tPhase3Calc/6(velDelta)  "<velDelta>"
  Bus Selector1/6 -> distPhase3Calc/7(accelMax)  "<accelMax>"
  Bus Selector1/5 -> distPhase3Calc/6(tPhase2)  "<tPhase2>"
  Bus Selector1/4 -> distPhase3Calc/5(tPhase1)  "<tPhase1>"
  Bus Selector1/3 -> distPhase3Calc/4(Kjerk)  "<Kjerk>"
  Bus Selector1/2 -> distPhase3Calc/3(subjLongAccelFilt)  "<subjLongAccelFilt>"
  Bus Selector1/1 -> distPhase3Calc/2(subjVel)  "<subjVel>"

== system TosAeb/FcwAebJa_Step_sys/ALG_AEBSystem/ALG_AEBController/AEB_JaObjCalcApp/JAVehTgtInfoCalc/JAVehCollisonAssessInfoCalc/JaVehCollisonAssessInfoCalc/VehDistBrakeFullCalc/distBrakeCalc ==
  distPhase1 : Inport  OutDataTypeStr=single
  distPhase2 : Inport  Port=2  OutDataTypeStr=single
  distPhase3 : Inport  Port=3  OutDataTypeStr=single
  Add : Sum  Inputs=3  OutDataTypeStr=single
  distBrake : Outport
  wires:
  distPhase2/1 -> Add/2
  distPhase1/1 -> Add/1
  distPhase3/1 -> Add/3
  Add/1 -> distBrake/1

== system TosAeb/FcwAebJa_Step_sys/ALG_AEBSystem/ALG_AEBController/AEB_JaObjCalcApp/JAVehTgtInfoCalc/JAVehCollisonAssessInfoCalc/JaVehCollisonAssessInfoCalc/VehDistBrakeFullCalc/tBrakeCalc ==
  tPhase3 : Inport  OutDataTypeStr=single
  tPhase2 : Inport  Port=2  OutDataTypeStr=single
  tPhase1 : Inport  Port=3  OutDataTypeStr=single
  Add : Sum  Inputs=3  OutDataTypeStr=single
  tBrake : Outport
  wires:
  tPhase2/1 -> Add/2
  tPhase3/1 -> Add/1
  tPhase1/1 -> Add/3
  Add/1 -> tBrake/1

== system TosAeb/FcwAebJa_Step_sys/ALG_AEBSystem/ALG_AEBController/AEB_JaObjCalcApp/JAVehTgtInfoCalc/JAVehCollisonAssessInfoCalc/JaVehCollisonAssessInfoCalc/VelAbsTgtCorrCalc/RadicalRelevantCalc ==
  dy : Inport  OutDataTypeStr=single
  dxFrontBumper : Inport  Port=2  OutDataTypeStr=single
  subjYawRate : Inport  Port=3  OutDataTypeStr=single
  Bus Creator : BusCreator  Inputs=2
  Math Function : Math  Operator=hypot  SignedPower=on  SaturateOnIntegerOverflow=on
  Product1 : Product  OutDataTypeStr=single
  Sincos : Trigonometry  Operator=sincos
  Trigonometric Function : Trigonometry  Operator=atan2
  radialVelRel : Outport
  sincosCoef : Outport  Port=2
  wires:
  subjYawRate/1 -> Product1/1
  Math Function/1 -> Product1/2  "dvRadial"
  Product1/1 -> radialVelRel/1  "radialVelRel"
  dy/1 -> Math Function/2, Trigonometric Function/1
  dxFrontBumper/1 -> Trigonometric Function/2, Math Function/1
  Trigonometric Function/1 -> Sincos/1
  Sincos/2 -> Bus Creator/2  "cosCoef"
  Bus Creator/1 -> sincosCoef/1  "sincosCoef"
  Sincos/1 -> Bus Creator/1  "sinCoef"

== system TosAeb/FcwAebJa_Step_sys/ALG_AEBSystem/ALG_AEBController/AEB_JaObjCalcApp/JAVehTgtInfoCalc/JAVehCollisonAssessInfoCalc/JaVehCollisonAssessInfoCalc/VelAbsTgtCorrCalc/RadicalToXYTrans ==
  velRadicalXY : Inport
  sincosCoef : Inport  Port=2
  subjVel : Inport  Port=3  OutDataTypeStr=single
  Add3 : Sum  Inputs=++-  OutDataTypeStr=single
  Add4 : Sum  OutDataTypeStr=single
  Bus Creator4 : BusCreator  Inputs=2
  Bus Selector10 : BusSelector  OutputSignals=velRadicalXY.velRadicalY
  Bus Selector11 : BusSelector  OutputSignals=sincosCoef.sinCoef
  Bus Selector12 : BusSelector  OutputSignals=velRadicalXY.velRadicalX
  Bus Selector13 : BusSelector  OutputSignals=sincosCoef.sinCoef
  Bus Selector14 : BusSelector  OutputSignals=velRadicalXY.velRadicalY
  Bus Selector15 : BusSelector  OutputSignals=sincosCoef.cosCoef
  Bus Selector8 : BusSelector  OutputSignals=velRadicalXY.velRadicalX
  Bus Selector9 : BusSelector  OutputSignals=sincosCoef.cosCoef
  Product{7..10} (4 alike) : Product  OutDataTypeStr=single
  vxAbsTargetCorr : Outport
  vyAbsTargetCorr : Outport  Port=2
  wires:
  Product8/1 -> Add3/3
  Bus Selector13/1 -> Product9/2  "<sinCoef>"
  Add3/1 -> vxAbsTargetCorr/1  "vxAbsTargetCorr"
  Product7/1 -> Add3/2
  Bus Selector14/1 -> Product10/1  "<velRadicalY>"
  Bus Selector11/1 -> Product8/2  "<sinCoef>"
  Bus Selector10/1 -> Product8/1  "<velRadicalY>"
  subjVel/1 -> Add3/1
  Add4/1 -> vyAbsTargetCorr/1  "vyAbsTargetCorr"
  Bus Creator4/1 -> Bus Selector15/1, Bus Selector14/1, Bus Selector13/1, Bus Selector12/1, Bus Selector11/1, Bus Selector10/1, Bus Selector9/1, Bus Selector8/1
  Bus Selector9/1 -> Product7/2  "<cosCoef>"
  Product10/1 -> Add4/2
  Bus Selector15/1 -> Product10/2  "<cosCoef>"
  Bus Selector8/1 -> Product7/1  "<velRadicalX>"
  Product9/1 -> Add4/1
  Bus Selector12/1 -> Product9/1  "<velRadicalX>"
  velRadicalXY/1 -> Bus Creator4/1
  sincosCoef/1 -> Bus Creator4/2

== system TosAeb/FcwAebJa_Step_sys/ALG_AEBSystem/ALG_AEBController/AEB_JaObjCalcApp/JAVehTgtInfoCalc/JAVehCollisonAssessInfoCalc/JaVehCollisonAssessInfoCalc/VelAbsTgtCorrCalc/XYToRadicalTrans ==
  LongLatVelRel : Inport
  radialVelRel : Inport  Port=2  OutDataTypeStr=single
  sincosCoef : Inport  Port=3
  Add : Sum  OutDataTypeStr=single
  Add1 : Sum  OutDataTypeStr=single
  Add2 : Sum  Inputs=+-  OutDataTypeStr=single
  Bus Creator2 : BusCreator  Inputs=2
  Bus Creator3 : BusCreator  Inputs=2
  Bus Selector : BusSelector  OutputSignals=LongLatVelRel.vx
  Bus Selector1 : BusSelector  OutputSignals=sincosCoef.cosCoef
  Bus Selector2 : BusSelector  OutputSignals=LongLatVelRel.vy
  Bus Selector3 : BusSelector  OutputSignals=sincosCoef.sinCoef
  Bus Selector4 : BusSelector  OutputSignals=LongLatVelRel.vy
  Bus Selector5 : BusSelector  OutputSignals=sincosCoef.cosCoef
  Bus Selector6 : BusSelector  OutputSignals=LongLatVelRel.vx
  Bus Selector7 : BusSelector  OutputSignals=sincosCoef.sinCoef
  Product{2,4..6} (4 alike) : Product  OutDataTypeStr=single
  velRadicalXY : Outport
  wires:
  Product6/1 -> Add2/2
  Bus Creator3/1 -> velRadicalXY/1  "velRadicalXY"
  Product5/1 -> Add2/1
  Bus Selector2/1 -> Product4/1  "<vy>"
  Product4/1 -> Add/2
  Product2/1 -> Add/1
  Bus Creator2/1 -> Bus Selector7/1, Bus Selector6/1, Bus Selector5/1, Bus Selector4/1, Bus Selector3/1, Bus Selector2/1, Bus Selector1/1, Bus Selector/1
  Bus Selector5/1 -> Product5/2  "<cosCoef>"
  Add1/1 -> Bus Creator3/2  "velRadicalY"
  Bus Selector6/1 -> Product6/1  "<vx>"
  Add/1 -> Bus Creator3/1  "velRadicalX"
  Bus Selector4/1 -> Product5/1  "<vy>"
  Bus Selector1/1 -> Product2/2  "<cosCoef>"
  radialVelRel/1 -> Add1/2
  Bus Selector3/1 -> Product4/2  "<sinCoef>"
  Bus Selector/1 -> Product2/1  "<vx>"
  Bus Selector7/1 -> Product6/2  "<sinCoef>"
  sincosCoef/1 -> Bus Creator2/2
  LongLatVelRel/1 -> Bus Creator2/1
  Add2/1 -> Add1/1

== system TosAeb/FcwAebJa_Step_sys/ALG_AEBSystem/ALG_AEBController/AEB_JaObjCalcApp/JAVehTgtInfoCalc/JAVehCollisonAssessInfoCalc/JaVehCollisonAssessInfoCalc/tBrakeingCalc/tBrakeCalc ==
  tBarke : Inport  OutDataTypeStr=single
  TTB : Outport
  wires:
  tBarke/1 -> TTB/1

== system TosAeb/FcwAebJa_Step_sys/ALG_AEBSystem/ALG_AEBController/AEB_JaObjCalcApp/JAVehTgtInfoCalc/JAVehCollisonAssessInfoCalc/JaVehCollisonAssessInfoCalc/tBrakeingCalc/tBrakeLookup ==
  subjVel : Inport
  1-D Lookup Table : Lookup_n-D  NumberOfTableDimensions=1  BreakpointsForDimension1=P_JaVehCA_BrkWorkTimeX  Table=P_JaVehCA_SubjVelBrkWorkTimeX  ExtrapMethod=Clip  InternalRulePriority=Speed  RndMeth=Simplest  InputSameDT=on
  TTB : Outport
  wires:
  1-D Lookup Table/1 -> TTB/1
  subjVel/1 -> 1-D Lookup Table/1

== system TosAeb/FcwAebJa_Step_sys/ALG_AEBSystem/ALG_AEBController/AEB_JaObjCalcApp/JaCycTgtInfoCalc/JaCycCollisonAssessInfo/JaCycCollisonAssessInfoCalc/PedDistBrakeFullCalc/BrkSys ==
  subjVel : Inport
  subjLongAccel : Inport  Port=2  OutDataTypeStr=single
  BrkJerkMaxFullBrakeLookupTable : Lookup_n-D  NumberOfTableDimensions=1  BreakpointsForDimension1=P_AEB_JaCycCA_BrkJerkMaxFullBrakeX  Table=P_AEB_JaCycCA_BrkJerkMaxFullBrakeY  ExtrapMethod=Clip  InternalRulePriority=Speed  RndMeth=Simplest  InputSameDT=on
  DecelMaxLookupTable : Lookup_n-D  NumberOfTableDimensions=1  BreakpointsForDimension1=P_AEB_JaCycCA_DecelMaxFullBrakeX  Table=P_AEB_JaCycCA_DecelMaxFullBrakeY  ExtrapMethod=Clip  InternalRulePriority=Speed  RndMeth=Simplest  InputSameDT=on
  MinMax : MinMax  Function=max  Inputs=2  OutDataTypeStr=single
  PKjerk : Constant  Commented=on  Value=P_AEB_JaPedCA_BrkJerkMaxFullBrake  OutDataTypeStr=single
  PaccelMax1 : Constant  Commented=on  Value=P_AEB_JaPedCA_DecelMaxFullBrake  OutDataTypeStr=single
  PtDelay : Constant  Value=P_AEB_JaCycCA_BrkSysDelayTimeFullBrake  OutDataTypeStr=single
  PvelDelta : Constant  Value=P_AEB_JaCycCA_VelReduceMaxFullBrake  OutDataTypeStr=single
  tDelay : Outport
  Kjerk : Outport  Port=2
  velDelta : Outport  Port=3
  accelMax : Outport  Port=4
  subjLongAccelFilt : Outport  Port=5
  wires:
  PtDelay/1 -> tDelay/1
  BrkJerkMaxFullBrakeLookupTable/1 -> Kjerk/1
  PvelDelta/1 -> velDelta/1
  DecelMaxLookupTable/1 -> accelMax/1, MinMax/1
  subjLongAccel/1 -> MinMax/2
  MinMax/1 -> subjLongAccelFilt/1
  subjVel/1 -> BrkJerkMaxFullBrakeLookupTable/1, DecelMaxLookupTable/1

== system TosAeb/FcwAebJa_Step_sys/ALG_AEBSystem/ALG_AEBController/AEB_JaObjCalcApp/JaCycTgtInfoCalc/JaCycCollisonAssessInfo/JaCycCollisonAssessInfoCalc/PedDistBrakeFullCalc/DistPhase1Calc ==
  subjVel : Inport  OutDataTypeStr=single
  tPhase1 : Inport  Port=2  OutDataTypeStr=single
  subjLongAccelFilt : Inport  Port=3  OutDataTypeStr=single
  Add : Sum  OutDataTypeStr=single
  Constant : Constant  Value=0.5  OutDataTypeStr=single
  Product : Product  Inputs=4  OutDataTypeStr=single
  Product1 : Product  OutDataTypeStr=single
  distPhase1 : Outport
  wires:
  tPhase1/1 -> Product1/2, Product/2, Product/1
  subjLongAccelFilt/1 -> Product/3
  Constant/1 -> Product/4
  subjVel/1 -> Product1/1
  Product1/1 -> Add/1
  Product/1 -> Add/2
  Add/1 -> distPhase1/1

== system TosAeb/FcwAebJa_Step_sys/ALG_AEBSystem/ALG_AEBController/AEB_JaObjCalcApp/JaCycTgtInfoCalc/JaCycCollisonAssessInfo/JaCycCollisonAssessInfoCalc/PedDistBrakeFullCalc/DistPhase2Calc ==
  subjVel : Inport  OutDataTypeStr=single
  Kjerk : Inport  Port=2  OutDataTypeStr=single
  tPhase1 : Inport  Port=3  OutDataTypeStr=single
  subjLongAccelFilt : Inport  Port=4  OutDataTypeStr=single
  accelMax : Inport  Port=5  OutDataTypeStr=single
  distPhase2Calc : Subsystem
  tPhase2Calc : Subsystem
  distPhase2 : Outport
  egoStopFlg : Outport  Port=2
  tJerkWork : Outport  Port=3
  tPhase2 : Outport  Port=4
  wires:
  subjLongAccelFilt/1 -> tPhase2Calc/3(subjLongAccelFilt), distPhase2Calc/3(subjLongAccelFilt)
  Kjerk/1 -> tPhase2Calc/2(Kjerk), distPhase2Calc/4(Kjerk)
  subjVel/1 -> distPhase2Calc/5(subjVel), tPhase2Calc/1(subjVel)
  accelMax/1 -> tPhase2Calc/4(accelMax)
  tPhase2Calc/3(tPhase2) -> tPhase2/1, distPhase2Calc/1(tPhase2)
  distPhase2Calc/1(distPhase2) -> distPhase2/1
  tPhase2Calc/2(tJerkWork) -> tJerkWork/1
  tPhase2Calc/1(egoStopFlg) -> egoStopFlg/1
  tPhase1/1 -> distPhase2Calc/2(tPhase1)

== system TosAeb/FcwAebJa_Step_sys/ALG_AEBSystem/ALG_AEBController/AEB_JaObjCalcApp/JaCycTgtInfoCalc/JaCycCollisonAssessInfo/JaCycCollisonAssessInfoCalc/PedDistBrakeFullCalc/DistPhase3Calc ==
  egoStopFlg : Inport
  tJerkWork : Inport  Port=2  OutDataTypeStr=single
  tPhase2 : Inport  Port=3  OutDataTypeStr=single
  tPhase1 : Inport  Port=4  OutDataTypeStr=single
  velDelta : Inport  Port=5  OutDataTypeStr=single
  subjVel : Inport  Port=6  OutDataTypeStr=single
  subjLongAccelFilt : Inport  Port=7  OutDataTypeStr=single
  Kjerk : Inport  Port=8  OutDataTypeStr=single
  accelMax : Inport  Port=9  OutDataTypeStr=single
  Bus Creator : BusCreator  Inputs=8
  Bus Selector : BusSelector  OutputSignals=subjVel,subjLongAccelFilt,tJerkWork,Kjerk,accelMax,velDelta
  Bus Selector1 : BusSelector  OutputSignals=subjVel,subjLongAccelFilt,Kjerk,tPhase1,tPhase2,accelMax
  If : If  IfExpression=u1
  Merge : Merge
  distPhase3Calc : Subsystem
  tPhase3Calc : Subsystem  TreatAsAtomicUnit=on
  tPhase3Calc1 : Subsystem  TreatAsAtomicUnit=on
  distPhase3 : Outport
  tPhase3 : Outport  Port=2
  wires:
  If/1 -> tPhase3Calc1/Action
  egoStopFlg/1 -> If/1
  If/2 -> tPhase3Calc/Action
  tPhase3Calc1/1(tPhase3) -> Merge/1
  tPhase3Calc/1(tPhase3) -> Merge/2
  Merge/1 -> tPhase3/1, distPhase3Calc/1(tPhase3)
  distPhase3Calc/1(distPhase3) -> distPhase3/1
  accelMax/1 -> Bus Creator/8  "accelMax"
  Kjerk/1 -> Bus Creator/7  "Kjerk"
  subjLongAccelFilt/1 -> Bus Creator/6  "subjLongAccelFilt"
  subjVel/1 -> Bus Creator/5  "subjVel"
  tJerkWork/1 -> Bus Creator/1  "tJerkWork"
  tPhase1/1 -> Bus Creator/2  "tPhase1"
  tPhase2/1 -> Bus Creator/3  "tPhase2"
  velDelta/1 -> Bus Creator/4  "velDelta"
  Bus Creator/1 -> Bus Selector1/1, Bus Selector/1
  Bus Selector/3 -> tPhase3Calc/3(tJerkWork)  "<tJerkWork>"
  Bus Selector/4 -> tPhase3Calc/4(Kjerk)  "<Kjerk>"
  Bus Selector/2 -> tPhase3Calc/2(subjLongAccelFilt)  "<subjLongAccelFilt>"
  Bus Selector/5 -> tPhase3Calc/5(accelMax)  "<accelMax>"
  Bus Selector/1 -> tPhase3Calc/1(subjVel)  "<subjVel>"
  Bus Selector/6 -> tPhase3Calc/6(velDelta)  "<velDelta>"
  Bus Selector1/6 -> distPhase3Calc/7(accelMax)  "<accelMax>"
  Bus Selector1/5 -> distPhase3Calc/6(tPhase2)  "<tPhase2>"
  Bus Selector1/4 -> distPhase3Calc/5(tPhase1)  "<tPhase1>"
  Bus Selector1/3 -> distPhase3Calc/4(Kjerk)  "<Kjerk>"
  Bus Selector1/2 -> distPhase3Calc/3(subjLongAccelFilt)  "<subjLongAccelFilt>"
  Bus Selector1/1 -> distPhase3Calc/2(subjVel)  "<subjVel>"

== system TosAeb/FcwAebJa_Step_sys/ALG_AEBSystem/ALG_AEBController/AEB_JaObjCalcApp/JaCycTgtInfoCalc/JaCycCollisonAssessInfo/JaCycCollisonAssessInfoCalc/PedDistBrakeFullCalc/distBrakeCalc ==
  distPhase1 : Inport  OutDataTypeStr=single
  distPhase2 : Inport  Port=2  OutDataTypeStr=single
  distPhase3 : Inport  Port=3  OutDataTypeStr=single
  Add : Sum  Inputs=3  OutDataTypeStr=single
  distBrake : Outport
  wires:
  distPhase2/1 -> Add/2
  distPhase1/1 -> Add/1
  distPhase3/1 -> Add/3
  Add/1 -> distBrake/1

== system TosAeb/FcwAebJa_Step_sys/ALG_AEBSystem/ALG_AEBController/AEB_JaObjCalcApp/JaCycTgtInfoCalc/JaCycCollisonAssessInfo/JaCycCollisonAssessInfoCalc/PedDistBrakeFullCalc/tBrakeCalc ==
  tPhase3 : Inport  OutDataTypeStr=single
  tPhase1 : Inport  Port=2  OutDataTypeStr=single
  tPhase2 : Inport  Port=3  OutDataTypeStr=single
  Add : Sum  Inputs=+++  OutDataTypeStr=single
  tBrake : Outport
  wires:
  tPhase2/1 -> Add/2
  tPhase3/1 -> Add/3
  tPhase1/1 -> Add/1
  Add/1 -> tBrake/1
